simulink model "wlan_phy_rx_pmd"  (Simulink 7.8)
settings: Solver=VariableStepDiscrete  SolverMode=SingleTasking  StartTime=0.0  StopTime=rx_sim_time  RelTol=1e-3
PostLoadFcn:
  | wlan_phy_rx_init
InitFcn:
  | wlan_phy_rx_init
StartFcn:
  | wlan_phy_rx_init
values from neighbouring files (symbols this model uses):
  barker_seq20 = 0.95 * circshift(barker_seq20, [0 4]) ./ max(abs(barker_seq20))    [wlan_phy_rx_init.m]
  bit_scrambler_lfsr_bytes = sum(bit_scrambler_lfsr_bytes .* repmat(2.^[0:7], 127, 1),2)    [wlan_phy_rx_init.m]
  deinterleave_ROM = [deinterleave_ROM interleave_11n_64QAM zeros(1, 512-length(interleave_11n_64QAM))]    [wlan_phy_rx_init.m]
  longCorr_coef_i = [3*real(longCorr_coef)]    [wlan_phy_rx_init.m]
  longCorr_coef_q = [3*imag(longCorr_coef)]    [wlan_phy_rx_init.m]
  MAX_CP_LEN = 32    [wlan_phy_rx_init.m]
  MAX_NCBPS = NCBPS_11n_64QAM    (= 312)    [wlan_phy_rx_init.m]
  MAX_NUM_BYTES = 2^16-1    (= 65535)    [wlan_phy_rx_init.m]
  MAX_NUM_SC = 64    [wlan_phy_rx_init.m]
  MAX_NUM_SYMS = 600    [wlan_phy_rx_init.m]
  NCBPS_11a_BPSK = 48    [wlan_phy_rx_init.m]
  NDBPS_11a_BPSK12 = 24    [wlan_phy_rx_init.m]
  PHY_SIGNAL_MIN_LEN = 14    [wlan_phy_rx_init.m]
  preFFT_sampBuff_numSamps = 4*MAX_NUM_SC    (= 256)    [wlan_phy_rx_init.m]
  rx_sim_sig_samp_time = 8    [wlan_phy_rx_init.m]
  scr_ind_rev = zeros(1,128)    [wlan_phy_rx_init.m]
undefined symbols (not defined in the file or in its folder): gcbh, mcs_rom_11ag, mcs_rom_11n, xlBlockLoadCallback, xlGetNormalizedPeriod, xlipgetpartsetting
libraries: xbsIndex_r4 x3862, simulink x1, xbsEDKLib_r4 x1
(part 12 of 23)

== system wlan_phy_rx_pmd/Sync & Antenna Sel/PHY CCA/S-R Latch3 ==
  S : Inport
  R : Inport  Port=2
  Constant1 : xbsIndex_r4/Constant  const=1  gui_display_data_type=Fixed-point  arith_type=Boolean  n_bits=1  bin_pt=0  preci_type=Single  exp_width=8  frac_width=24  explicit_period=off  period=1  equ=P=C  opselect=C  inp2=PCIN>>17  opr=+  inp1=P  carry=CIN  dbl_ovrd=off  block_type=constant
      settings: block_version=VER_STRING_GOES_HERE
  Convert1 : xbsIndex_r4/Convert  gui_display_data_type=Boolean  arith_type=Boolean  n_bits=1  bin_pt=0  float_type=Single  exp_bits=8  fraction_bits=24  quantization=Truncate  overflow=Wrap  en=off  latency=0  dbl_ovrd=off  pipeline=off  block_type=convert
  Convert2 : xbsIndex_r4/Convert  gui_display_data_type=Boolean  arith_type=Boolean  n_bits=1  bin_pt=0  float_type=Single  exp_bits=8  fraction_bits=24  quantization=Truncate  overflow=Wrap  en=off  latency=0  dbl_ovrd=off  pipeline=off  block_type=convert
  Register2 : xbsIndex_r4/Register  init=0  rst=on  en=on  dbl_ovrd=off  block_type=register
      settings: xl_area=[1 1 0 0 0 0 0]  block_version=VER_STRING_GOES_HERE
  Q : Outport
  wires:
  Constant1/1 -> Register2/1
  R/1 -> Convert1/1
  Register2/1 -> Q/1
  S/1 -> Convert2/1
  Convert1/1 -> Register2/2
  Convert2/1 -> Register2/3

== system wlan_phy_rx_pmd/Sync & Antenna Sel/Pkt Det/DSSS Ant Sel Logic ==
  Det A : Inport
  Det B : Inport  Port=2
  Det C : Inport  Port=3
  Det D : Inport  Port=4
  Concat : xbsIndex_r4/Concat  num_inputs=4  dbl_ovrd=off  block_type=concat
  Logical{,2..4} (4 alike) : xbsIndex_r4/Logical  logical_function=AND  inputs=2  en=off  latency=0  precision=Full  arith_type=Unsigned  n_bits=16  bin_pt=0  align_bp=on  dbl_ovrd=off  block_type=logical
  Logical1 : xbsIndex_r4/Logical  logical_function=OR  inputs=4  en=off  latency=0  precision=Full  arith_type=Unsigned  n_bits=16  bin_pt=0  align_bp=on  dbl_ovrd=off  block_type=logical
  Pkt Det : Outport
  tag scope: PKTDET_STATUS_DSSS=global
  wires:
  Logical4/1 -> Logical1/4, Concat/4
  Logical3/1 -> Logical1/3, Concat/3
  Det D/1 -> Logical4/2
  Det C/1 -> Logical3/2
  [regRx_pktDetEn_RFD] -> Logical4/1
  [regRx_pktDetEn_RFC] -> Logical3/1
  [regRx_pktDetEn_RFB] -> Logical2/1
  Det B/1 -> Logical2/2
  Det A/1 -> Logical/2
  Logical1/1 -> Pkt Det/1
  Logical2/1 -> Logical1/2, Concat/2
  Logical/1 -> Logical1/1, Concat/1
  [regRx_pktDetEn_RFA] -> Logical/1
  Concat/1 -> [PKTDET_STATUS_DSSS]

== system wlan_phy_rx_pmd/Sync & Antenna Sel/Pkt Det/Ext Pkt Det ==
  Reset : Inport
  Constant : Constant  Value=0
  Convert : xbsIndex_r4/Convert  gui_display_data_type=Boolean  arith_type=Boolean  n_bits=16  bin_pt=14  float_type=Single  exp_bits=8  fraction_bits=24  quantization=Truncate  overflow=Wrap  en=off  latency=0  dbl_ovrd=off  pipeline=off  block_type=convert
  Convert1 : xbsIndex_r4/Convert  gui_display_data_type=Boolean  arith_type=Boolean  n_bits=16  bin_pt=14  float_type=Single  exp_bits=8  fraction_bits=24  quantization=Truncate  overflow=Wrap  en=off  latency=0  dbl_ovrd=off  pipeline=off  block_type=convert
  Convert2 : xbsIndex_r4/Convert  gui_display_data_type=Boolean  arith_type=Boolean  n_bits=16  bin_pt=14  float_type=Single  exp_bits=8  fraction_bits=24  quantization=Truncate  overflow=Wrap  en=off  latency=0  dbl_ovrd=off  pipeline=off  block_type=convert
  Debouce : Subsystem
  Logical1 : xbsIndex_r4/Logical  logical_function=AND  inputs=2  en=off  latency=0  precision=Full  arith_type=Unsigned  n_bits=16  bin_pt=0  align_bp=on  dbl_ovrd=off  block_type=logical
  Posedge1 : Subsystem
  Register{1..5} (5 alike) : xbsIndex_r4/Register  init=0  rst=off  en=off  dbl_ovrd=off  block_type=register
  S-R Latch : Subsystem
  pkt_det_in : xbsIndex_r4/Gateway In  gui_display_data_type=Fixed-point  arith_type=Unsigned  n_bits=1  bin_pt=0  preci_type=Single  exp_width=8  frac_width=24  quantization="Round  (unbiased: +/- Inf)"  overflow=Saturate  period=1  dbl_ovrd=off  timing_constraint=None  locs_specified=off  LOCs={}  UseAsADC=off  ADCChannel='1'  inherit_from_input=off  hdl_port=on  block_type=gatewayin
  Det : Outport
  wires:
  Constant/1 -> pkt_det_in/1
  S-R Latch/1(Q) -> Logical1/2
  Posedge1/1(Q) -> Convert1/1
  Reset/1 -> Register5/1
  Debouce/1(Q) -> Posedge1/1(D)
  pkt_det_in/1 -> Register4/1
  Logical1/1 -> Det/1
  [regRx_pktDetEn_Ext] -> Convert2/1
  Convert/1 -> S-R Latch/1(R)
  Convert1/1 -> S-R Latch/2(S)
  Convert2/1 -> Logical1/1
  Register4/1 -> Register1/1
  Register1/1 -> Register2/1
  Register2/1 -> Debouce/1(D)
  Register3/1 -> Debouce/2(R), Convert/1
  Register5/1 -> Register3/1

== system wlan_phy_rx_pmd/Sync & Antenna Sel/Pkt Det/OFDM Ant Sel Logic ==
  Ext Det : Inport
  Det A : Inport  Port=2
  Det B : Inport  Port=3
  Det C : Inport  Port=4
  Det D : Inport  Port=5
  Concat : xbsIndex_r4/Concat  num_inputs=5  dbl_ovrd=off  block_type=concat
  Logical{,2..4} (4 alike) : xbsIndex_r4/Logical  logical_function=AND  inputs=2  en=off  latency=0  precision=Full  arith_type=Unsigned  n_bits=16  bin_pt=0  align_bp=on  dbl_ovrd=off  block_type=logical
  Logical1 : xbsIndex_r4/Logical  logical_function=OR  inputs=4  en=off  latency=0  precision=Full  arith_type=Unsigned  n_bits=16  bin_pt=0  align_bp=on  dbl_ovrd=off  block_type=logical
  Pkt Det : Outport
  tag scope: PKTDET_STATUS_OFDM=global
  wires:
  [regRx_pktDetEn_RFA] -> Logical/1
  Logical/1 -> Logical1/1, Concat/5
  Logical2/1 -> Logical1/2, Concat/4
  Logical1/1 -> Pkt Det/1
  Det A/1 -> Logical/2
  Det B/1 -> Logical2/2
  [regRx_pktDetEn_RFB] -> Logical2/1
  [regRx_pktDetEn_RFC] -> Logical3/1
  [regRx_pktDetEn_RFD] -> Logical4/1
  Det C/1 -> Logical3/2
  Det D/1 -> Logical4/2
  Logical3/1 -> Logical1/3, Concat/3
  Logical4/1 -> Logical1/4, Concat/2
  Concat/1 -> [PKTDET_STATUS_OFDM]
  Ext Det/1 -> Concat/1

== system wlan_phy_rx_pmd/Sync & Antenna Sel/Pkt Det/RF A Det ==
  Reset OFDM : Inport
  Reset DSSS : Inport  Port=2
  Rx I : Inport  Port=3
  Rx Q : Inport  Port=4
  IQ Valid : Inport  Port=5
  RSSI : Inport  Port=6
  Auto-Corr Pkt Det : Subsystem
  Logical : xbsIndex_r4/Logical  logical_function=OR  inputs=2  en=off  latency=0  precision=Full  arith_type=Unsigned  n_bits=16  bin_pt=0  align_bp=on  dbl_ovrd=off  block_type=logical
  Logical1 : xbsIndex_r4/Logical  logical_function=OR  inputs=2  en=off  latency=0  precision=Full  arith_type=Unsigned  n_bits=16  bin_pt=0  align_bp=on  dbl_ovrd=off  block_type=logical
  Logical2 : xbsIndex_r4/Logical  logical_function=AND  inputs=2  en=off  latency=0  precision=Full  arith_type=Unsigned  n_bits=16  bin_pt=0  align_bp=on  dbl_ovrd=off  block_type=logical
  Logical3 : xbsIndex_r4/Logical  logical_function=AND  inputs=2  en=off  latency=0  precision=Full  arith_type=Unsigned  n_bits=16  bin_pt=0  align_bp=on  dbl_ovrd=off  block_type=logical
  Mux : xbsIndex_r4/Mux  inputs=2  en=off  latency=0  precision=Full  arith_type=Unsigned  n_bits=16  bin_pt=14  quantization=Truncate  overflow=Wrap  dbl_ovrd=off  block_type=mux
  Mux1 : xbsIndex_r4/Mux  inputs=2  en=off  latency=0  precision=Full  arith_type=Unsigned  n_bits=16  bin_pt=14  quantization=Truncate  overflow=Wrap  dbl_ovrd=off  block_type=mux
  RSSI Pkt Det : Subsystem
  Det OFDM : Outport
  Det DSSS : Outport  Port=2
  wires:
  Logical/1 -> Mux/2
  IQ Valid/1 -> Auto-Corr Pkt Det/5(rx_valid)
  Rx Q/1 -> Auto-Corr Pkt Det/4(rx_Q)
  Rx I/1 -> Auto-Corr Pkt Det/3(rx_I)
  Logical1/1 -> Mux1/2
  Reset OFDM/1 -> Auto-Corr Pkt Det/1(Reset OFDM), RSSI Pkt Det/1(Reset OFDM)
  Reset DSSS/1 -> Auto-Corr Pkt Det/2(Reset DSSS), RSSI Pkt Det/2(Reset DSSS)
  [regRx_pktDet_requireBothA] -> Mux/1, Mux1/1
  Logical3/1 -> Mux/3
  Mux/1 -> Det OFDM/1
  RSSI/1 -> RSSI Pkt Det/3(Avg RSSI)
  Auto-Corr Pkt Det/2(Det DSSS) -> Logical1/1, Logical2/1
  RSSI Pkt Det/2(PktDet DSSS) -> Logical1/2, Logical2/2
  Auto-Corr Pkt Det/1(Det OFDM) -> Logical/1, Logical3/1
  RSSI Pkt Det/1(PktDet OFDM) -> Logical/2, Logical3/2
  Logical2/1 -> Mux1/3
  Mux1/1 -> Det DSSS/1

== system wlan_phy_rx_pmd/Sync & Antenna Sel/Pkt Det/RF B Det ==
  Reset OFDM : Inport
  Reset DSSS : Inport  Port=2
  Rx I : Inport  Port=3
  Rx Q : Inport  Port=4
  IQ Valid : Inport  Port=5
  RSSI : Inport  Port=6
  Auto-Corr Pkt Det : Subsystem
  Logical : xbsIndex_r4/Logical  logical_function=OR  inputs=2  en=off  latency=0  precision=Full  arith_type=Unsigned  n_bits=16  bin_pt=0  align_bp=on  dbl_ovrd=off  block_type=logical
  Logical1 : xbsIndex_r4/Logical  logical_function=OR  inputs=2  en=off  latency=0  precision=Full  arith_type=Unsigned  n_bits=16  bin_pt=0  align_bp=on  dbl_ovrd=off  block_type=logical
  Logical2 : xbsIndex_r4/Logical  logical_function=AND  inputs=2  en=off  latency=0  precision=Full  arith_type=Unsigned  n_bits=16  bin_pt=0  align_bp=on  dbl_ovrd=off  block_type=logical
  Logical3 : xbsIndex_r4/Logical  logical_function=AND  inputs=2  en=off  latency=0  precision=Full  arith_type=Unsigned  n_bits=16  bin_pt=0  align_bp=on  dbl_ovrd=off  block_type=logical
  Mux : xbsIndex_r4/Mux  inputs=2  en=off  latency=0  precision=Full  arith_type=Unsigned  n_bits=16  bin_pt=14  quantization=Truncate  overflow=Wrap  dbl_ovrd=off  block_type=mux
  Mux1 : xbsIndex_r4/Mux  inputs=2  en=off  latency=0  precision=Full  arith_type=Unsigned  n_bits=16  bin_pt=14  quantization=Truncate  overflow=Wrap  dbl_ovrd=off  block_type=mux
  RSSI Pkt Det : Subsystem
  Det OFDM : Outport
  Det DSSS : Outport  Port=2
  wires:
  RSSI Pkt Det/1(PktDet OFDM) -> Logical3/2, Logical/2
  Auto-Corr Pkt Det/1(Det OFDM) -> Logical3/1, Logical/1
  RSSI Pkt Det/2(PktDet DSSS) -> Logical1/2, Logical2/2
  Auto-Corr Pkt Det/2(Det DSSS) -> Logical1/1, Logical2/1
  RSSI/1 -> RSSI Pkt Det/3(Avg RSSI)
  Mux/1 -> Det OFDM/1
  Logical3/1 -> Mux/3
  [regRx_pktDet_requireBothB] -> Mux/1, Mux1/1
  Reset DSSS/1 -> RSSI Pkt Det/2(Reset DSSS), Auto-Corr Pkt Det/2(Reset DSSS)
  Reset OFDM/1 -> RSSI Pkt Det/1(Reset OFDM), Auto-Corr Pkt Det/1(Reset OFDM)
  Rx I/1 -> Auto-Corr Pkt Det/3(rx_I)
  Rx Q/1 -> Auto-Corr Pkt Det/4(rx_Q)
  IQ Valid/1 -> Auto-Corr Pkt Det/5(rx_valid)
  Logical/1 -> Mux/2
  Mux1/1 -> Det DSSS/1
  Logical1/1 -> Mux1/2
  Logical2/1 -> Mux1/3

== system wlan_phy_rx_pmd/Sync & Antenna Sel/Resets/Negedge ==
  D : Inport
  Delay : xbsIndex_r4/Delay  rst=off  infoeditControl=Selection of Reset will increase slice count due to use of real FFs and instead of SRLs  en=off  latency=1  dbl_ovrd=off  reg_retiming=off  block_type=delay
      settings: infoedit=Hardware notes: A delay line is a chain, each link of which is an SRL16 followed by a flip-flop. If register retiming is enabled, the delay line is a chain of flip-flops.  xl_area=[1 1 0 0 0 0 0]  block_version=VER_STRING_GOES_HERE
  Inverter : xbsIndex_r4/Inverter  en=off  latency=0  dbl_ovrd=off  block_type=inv
      settings: xl_area=[0 0 0 0 0 0 0]  block_version=VER_STRING_GOES_HERE
  Logical1 : xbsIndex_r4/Logical  logical_function=AND  inputs=2  en=off  latency=0  precision=Full  arith_type=Unsigned  n_bits=8  bin_pt=2  align_bp=on  dbl_ovrd=off  block_type=logical
      settings: xl_area=[1 0 0 1 0 0 0]  block_version=VER_STRING_GOES_HERE
  Q : Outport
  wires:
  D/1 -> Delay/1, Inverter/1
  Logical1/1 -> Q/1
  Delay/1 -> Logical1/1
  Inverter/1 -> Logical1/2

== system wlan_phy_rx_pmd/Sync & Antenna Sel/Resets/Reset Gen ==
  note: Extend pkt det reset by programmable
      | duration, to avoid false positives after
      | actual receptions due to Rx transients
      | during AGC reset
  Done : Inport
  Concat : xbsIndex_r4/Concat  num_inputs=2  dbl_ovrd=off  block_type=concat
  Constant : xbsIndex_r4/Constant  const=0  gui_display_data_type=Fixed-point  arith_type=Unsigned  n_bits=4  bin_pt=0  preci_type=Single  exp_width=8  frac_width=24  explicit_period=off  period=1  equ=P=C  opselect=C  inp2=PCIN>>17  opr=+  inp1=P  carry=CIN  dbl_ovrd=off  block_type=constant
  Counter1 : xbsIndex_r4/Counter  cnt_type=Free Running  cnt_to=Inf  operation=Up  start_count=0  cnt_by_val=1  arith_type=Unsigned  n_bits=10  bin_pt=0  load_pin=off  rst=on  en=on  explicit_period=off  period=4  dbl_ovrd=off  use_behavioral_HDL=off  implementation=Fabric  use_rpm=off  block_type=counter
  Inverter : xbsIndex_r4/Inverter  en=off  latency=0  dbl_ovrd=off  block_type=inv
      settings: xl_area=[0 0 0 0 0 0 0]  block_version=VER_STRING_GOES_HERE
  Logical2 : xbsIndex_r4/Logical  logical_function=OR  inputs=3  en=off  latency=0  precision=Full  arith_type=Unsigned  n_bits=16  bin_pt=0  align_bp=on  dbl_ovrd=off  block_type=logical
  Register : xbsIndex_r4/Register  init=0  rst=off  en=off  dbl_ovrd=off  block_type=register
  Relational3 : xbsIndex_r4/Relational  mode=a>b  en=off  latency=0  dbl_ovrd=off  block_type=relational
      settings: block_version=VER_STRING_GOES_HERE
  S-R Latch2 : Subsystem
  negedge : Subsystem
  Reset : Outport
  wires:
  Done/1 -> Logical2/2, negedge/1(D)
  negedge/1(Q) -> S-R Latch2/1(S), Logical2/3
  S-R Latch2/1(Q) -> Counter1/2, Logical2/1, Inverter/1
  Counter1/1 -> Relational3/1
  Relational3/1 -> S-R Latch2/2(R)
  Logical2/1 -> Reset/1
  Concat/1 -> Relational3/2
  Constant/1 -> Concat/2
  [regRx_PKT_DET_RESET_EXT_DUR] -> Register/1
  Register/1 -> Concat/1
  Inverter/1 -> Counter1/1

== system wlan_phy_rx_pmd/Sync & Antenna Sel/Resets/Reset Gen1 ==
  note: Extend pkt det reset by programmable
      | duration, to avoid false positives after
      | actual receptions due to Rx transients
      | during AGC reset
  Done : Inport
  Concat : xbsIndex_r4/Concat  num_inputs=2  dbl_ovrd=off  block_type=concat
  Constant : xbsIndex_r4/Constant  const=0  gui_display_data_type=Fixed-point  arith_type=Unsigned  n_bits=4  bin_pt=0  preci_type=Single  exp_width=8  frac_width=24  explicit_period=off  period=1  equ=P=C  opselect=C  inp2=PCIN>>17  opr=+  inp1=P  carry=CIN  dbl_ovrd=off  block_type=constant
  Counter1 : xbsIndex_r4/Counter  cnt_type=Free Running  cnt_to=Inf  operation=Up  start_count=0  cnt_by_val=1  arith_type=Unsigned  n_bits=10  bin_pt=0  load_pin=off  rst=on  en=on  explicit_period=off  period=4  dbl_ovrd=off  use_behavioral_HDL=off  implementation=Fabric  use_rpm=off  block_type=counter
  Inverter : xbsIndex_r4/Inverter  en=off  latency=0  dbl_ovrd=off  block_type=inv
      settings: xl_area=[0 0 0 0 0 0 0]  block_version=VER_STRING_GOES_HERE
  Logical2 : xbsIndex_r4/Logical  logical_function=OR  inputs=3  en=off  latency=0  precision=Full  arith_type=Unsigned  n_bits=16  bin_pt=0  align_bp=on  dbl_ovrd=off  block_type=logical
  Register : xbsIndex_r4/Register  init=0  rst=off  en=off  dbl_ovrd=off  block_type=register
  Relational3 : xbsIndex_r4/Relational  mode=a>b  en=off  latency=0  dbl_ovrd=off  block_type=relational
      settings: block_version=VER_STRING_GOES_HERE
  S-R Latch2 : Subsystem
  negedge : Subsystem
  Reset : Outport
  wires:
  Inverter/1 -> Counter1/1
  Register/1 -> Concat/1
  [regRx_PKT_DET_RESET_EXT_DUR] -> Register/1
  Constant/1 -> Concat/2
  Concat/1 -> Relational3/2
  Logical2/1 -> Reset/1
  Relational3/1 -> S-R Latch2/2(R)
  Counter1/1 -> Relational3/1
  S-R Latch2/1(Q) -> Inverter/1, Logical2/1, Counter1/2
  negedge/1(Q) -> Logical2/3, S-R Latch2/1(S)
  Done/1 -> negedge/1(D), Logical2/2

== system wlan_phy_rx_pmd/CFO & Samp Buffer/CFO Correction/Sim Reset/Subsystem ==
  Disregard Subsystem : xbsIndex_r4/Disregard Subsystem  Tag=discardX  block_type=disregard
  Gateway In : xbsIndex_r4/Gateway In  gui_display_data_type=Boolean  arith_type=Boolean  n_bits=16  bin_pt=14  preci_type=Single  exp_width=8  frac_width=24  quantization="Round  (unbiased: +/- Inf)"  overflow=Saturate  period=1  dbl_ovrd=off  timing_constraint=None  locs_specified=off  LOCs={}  UseAsADC=off  ADCChannel='1'  inherit_from_input=off  hdl_port=on  block_type=gatewayin
  Pulse Generator : DiscretePulseGenerator  Period=1e9  PulseWidth=16  PhaseDelay=1
  Out1 : Outport
  wires:
  Pulse Generator/1 -> Gateway In/1
  Gateway In/1 -> Out1/1

== system wlan_phy_rx_pmd/CFO & Samp Buffer/Samp Buffer/FFT Load Ctrl/Count-To Calc ==
  Skip Sel : Inport
  AddSub1 : xbsIndex_r4/AddSub  mode=Addition  use_carryin=off  use_carryout=off  en=off  latency=0  precision=User Defined  arith_type=Unsigned  n_bits=ceil(log2(preFFT_sampBuff_numSamps))  bin_pt=0  quantization=Truncate  overflow=Wrap  dbl_ovrd=off  use_behavioral_HDL=off  hw_selection=Fabric  pipelined=off  block_type=addsub
  Constant4 : xbsIndex_r4/Constant  const=0  gui_display_data_type=Fixed-point  arith_type=Unsigned  n_bits=1  bin_pt=0  preci_type=Single  exp_width=8  frac_width=24  explicit_period=off  period=8  equ=P=C  opselect=C  inp2=PCIN>>17  opr=+  inp1=P  carry=CIN  dbl_ovrd=off  block_type=constant
  Display : Display
  Display1 : Display
  Gateway Out : xbsIndex_r4/Gateway Out  inherit_from_input=off  hdl_port=off  timing_constraint=None  locs_specified=off  LOCs={}  UseAsDAC=off  DACChannel='1'  block_type=gatewayout
  Gateway Out1 : xbsIndex_r4/Gateway Out  inherit_from_input=off  hdl_port=off  timing_constraint=None  locs_specified=off  LOCs={}  UseAsDAC=off  DACChannel='1'  block_type=gatewayout
  Mux : xbsIndex_r4/Mux  inputs=2  en=off  latency=0  precision=Full  arith_type=Unsigned  n_bits=16  bin_pt=14  quantization=Truncate  overflow=Wrap  dbl_ovrd=off  block_type=mux
  Samp+Skip : Outport
  Samp : Outport  Port=2
  wires:
  [regRx_CP_LEN] -> Mux/3, Gateway Out/1
  Skip Sel/1 -> Mux/1
  Constant4/1 -> Mux/2
  Mux/1 -> AddSub1/1
  AddSub1/1 -> Samp+Skip/1
  [regRx_NUM_SC] -> AddSub1/2, Samp/1, Gateway Out1/1
  Gateway Out/1 -> Display/1
  Gateway Out1/1 -> Display1/1

== system wlan_phy_rx_pmd/CFO & Samp Buffer/Samp Buffer/FFT Load Ctrl/First Samp Calc ==
  Wr Addr : Inport
  LTS Sync : Inport  Port=2
  AddSub2 : xbsIndex_r4/AddSub  mode=Subtraction  use_carryin=off  use_carryout=off  en=off  latency=0  precision=User Defined  arith_type=Unsigned  n_bits=ceil(log2(preFFT_sampBuff_numSamps))  bin_pt=0  quantization=Truncate  overflow=Wrap  dbl_ovrd=off  use_behavioral_HDL=off  hw_selection=Fabric  pipelined=off  block_type=addsub
  AddSub3 : xbsIndex_r4/AddSub  mode=Addition  use_carryin=off  use_carryout=off  en=off  latency=0  precision=User Defined  arith_type=Unsigned  n_bits=ceil(log2(preFFT_sampBuff_numSamps))  bin_pt=0  quantization=Truncate  overflow=Wrap  dbl_ovrd=off  use_behavioral_HDL=off  hw_selection=Fabric  pipelined=off  block_type=addsub
  Register : xbsIndex_r4/Register  init=0  rst=off  en=on  dbl_ovrd=off  block_type=register
  Shift : xbsIndex_r4/Shift  shift_dir=Left  shift_bits=1  en=off  latency=0  precision=Full  arith_type="Signed  (2's comp)"  n_bits=16  bin_pt=14  quantization=Truncate  overflow=Wrap  dbl_ovrd=off  block_type=shift
  Samp Addr : Outport
  wires:
  [regRx_NUM_SC] -> Shift/1
  Shift/1 -> AddSub3/1
  AddSub3/1 -> AddSub2/2
  Register/1 -> AddSub2/1
  Wr Addr/1 -> Register/1
  AddSub2/1 -> Samp Addr/1
  LTS Sync/1 -> Register/2
  [regRx_FFT_OFFSET] -> AddSub3/2

== system wlan_phy_rx_pmd/CFO & Samp Buffer/Samp Buffer/FFT Load Ctrl/Posedge ==
  note: Rising Edge Detection
  D : Inport
  Delay : xbsIndex_r4/Delay  rst=off  infoeditControl=Selection of Reset will increase slice count due to use of real FFs and instead of SRLs  en=off  latency=1  dbl_ovrd=off  reg_retiming=off  block_type=delay
      settings: infoedit=Hardware notes: A delay line is a chain, each link of which is an SRL16 followed by a flip-flop. If register retiming is enabled, the delay line is a chain of flip-flops.  xl_area=[1 1 0 0 0 0 0]  block_version=VER_STRING_GOES_HERE
  Inverter : xbsIndex_r4/Inverter  en=off  latency=0  dbl_ovrd=off  block_type=inv
      settings: xl_area=[0 0 0 0 0 0 0]  block_version=VER_STRING_GOES_HERE
  Logical1 : xbsIndex_r4/Logical  logical_function=AND  inputs=2  en=off  latency=0  precision=Full  arith_type=Unsigned  n_bits=8  bin_pt=2  align_bp=on  dbl_ovrd=off  block_type=logical
      settings: xl_area=[1 0 0 1 0 0 0]  block_version=VER_STRING_GOES_HERE
  Q : Outport
  wires:
  D/1 -> Logical1/2, Delay/1
  Logical1/1 -> Q/1
  Inverter/1 -> Logical1/1
  Delay/1 -> Inverter/1

== system wlan_phy_rx_pmd/CFO & Samp Buffer/Samp Buffer/FFT Load Ctrl/S-R Latch1 ==
  S : Inport
  R : Inport  Port=2
  Constant1 : xbsIndex_r4/Constant  const=1  gui_display_data_type=Fixed-point  arith_type=Boolean  n_bits=1  bin_pt=0  preci_type=Single  exp_width=8  frac_width=24  explicit_period=off  period=1  equ=P=C  opselect=C  inp2=PCIN>>17  opr=+  inp1=P  carry=CIN  dbl_ovrd=off  block_type=constant
      settings: block_version=VER_STRING_GOES_HERE
  Convert1 : xbsIndex_r4/Convert  gui_display_data_type=Boolean  arith_type=Boolean  n_bits=1  bin_pt=0  float_type=Single  exp_bits=8  fraction_bits=24  quantization=Truncate  overflow=Wrap  en=off  latency=0  dbl_ovrd=off  pipeline=off  block_type=convert
  Convert2 : xbsIndex_r4/Convert  gui_display_data_type=Boolean  arith_type=Boolean  n_bits=1  bin_pt=0  float_type=Single  exp_bits=8  fraction_bits=24  quantization=Truncate  overflow=Wrap  en=off  latency=0  dbl_ovrd=off  pipeline=off  block_type=convert
  Register2 : xbsIndex_r4/Register  init=0  rst=on  en=on  dbl_ovrd=off  block_type=register
      settings: xl_area=[1 1 0 0 0 0 0]  block_version=VER_STRING_GOES_HERE
  Q : Outport
  wires:
  S/1 -> Convert2/1
  Register2/1 -> Q/1
  R/1 -> Convert1/1
  Constant1/1 -> Register2/1
  Convert1/1 -> Register2/2
  Convert2/1 -> Register2/3

== system wlan_phy_rx_pmd/Chan Est & EQ/Equalizer, Pilot Processing & PHY Mode Det/PHY Mode Det/Mod Det ==
  note: PHY Mode Det calc = sum(abs(I) - abs(Q)):
      |   Large Positive => BPSK
      |   Large Negative => QBPSK
      |   Near Zero => QAM
  I : Inport
  Q : Inport  Port=2
  " BPSK" : xbsIndex_r4/Logical  logical_function=AND  inputs=2  en=off  latency=0  precision=Full  arith_type=Unsigned  n_bits=16  bin_pt=0  align_bp=on  dbl_ovrd=off  block_type=logical
  " QAM" : xbsIndex_r4/Inverter  en=off  latency=0  dbl_ovrd=off  block_type=inv
  " QBPSK" : xbsIndex_r4/Logical  logical_function=AND  inputs=2  en=off  latency=0  precision=Full  arith_type=Unsigned  n_bits=16  bin_pt=0  align_bp=on  dbl_ovrd=off  block_type=logical
  Absolute2 : xbsIndex_r4/Absolute  precision=Full  arith_type="Signed  (2's comp)"  n_bits=8  bin_pt=7  quantization=Truncate  overflow=Saturate  en=off  latency=0  dbl_ovrd=off  block_type=abs
  AddSub : xbsIndex_r4/AddSub  mode=Subtraction  use_carryin=off  use_carryout=off  en=off  latency=0  precision=Full  arith_type=Unsigned  n_bits=16  bin_pt=14  quantization=Truncate  overflow=Wrap  dbl_ovrd=off  use_behavioral_HDL=off  hw_selection=Fabric  pipelined=off  block_type=addsub
  Constant5 : xbsIndex_r4/Constant  const=0  gui_display_data_type=Fixed-point  arith_type=Unsigned  n_bits=2  bin_pt=0  preci_type=Single  exp_width=8  frac_width=24  explicit_period=off  period=1  equ=P=C  opselect=C  inp2=PCIN>>17  opr=+  inp1=P  carry=CIN  dbl_ovrd=off  block_type=constant
  Constant6 : xbsIndex_r4/Constant  const=20  gui_display_data_type=Fixed-point  arith_type=Unsigned  n_bits=5  bin_pt=0  preci_type=Single  exp_width=8  frac_width=24  explicit_period=off  period=1  equ=P=C  opselect=C  inp2=PCIN>>17  opr=+  inp1=P  carry=CIN  dbl_ovrd=off  block_type=constant
  Inverter : xbsIndex_r4/Inverter  en=off  latency=0  dbl_ovrd=off  block_type=inv
  Relational4 : xbsIndex_r4/Relational  mode=a>b  en=off  latency=1  dbl_ovrd=off  block_type=relational
  Relational5 : xbsIndex_r4/Relational  mode=a>b  en=off  latency=1  dbl_ovrd=off  block_type=relational
  QAM : Outport
  QBPSK : Outport  Port=2
  BPSK : Outport  Port=3
  wires:
  Constant5/1 -> Relational4/2
  AddSub/1 -> Relational4/1, Absolute2/1
  Absolute2/1 -> Relational5/1
  Constant6/1 -> Relational5/2
  Relational5/1 -> " BPSK"/1, " QBPSK"/1, " QAM"/1
  Relational4/1 -> " BPSK"/2, Inverter/1
  Inverter/1 -> " QBPSK"/2
  " BPSK"/1 -> BPSK/1
  I/1 -> AddSub/1
  Q/1 -> AddSub/2
  " QBPSK"/1 -> QBPSK/1
  " QAM"/1 -> QAM/1

== system wlan_phy_rx_pmd/Chan Est & EQ/Equalizer, Pilot Processing & PHY Mode Det/PHY Mode Det/edge ==
  D : Inport
  Delay6 : xbsIndex_r4/Delay  rst=off  infoeditControl=Selection of Reset will increase slice count due to use of real FFs and instead of SRLs  en=off  latency=1  dbl_ovrd=off  reg_retiming=off  block_type=delay
  Logical : xbsIndex_r4/Logical  logical_function=XOR  inputs=2  en=off  latency=0  precision=Full  arith_type=Unsigned  n_bits=16  bin_pt=0  align_bp=on  dbl_ovrd=off  block_type=logical
  Q : Outport
  wires:
  Delay6/1 -> Logical/2
  D/1 -> Logical/1, Delay6/1
  Logical/1 -> Q/1

== system wlan_phy_rx_pmd/Chan Est & EQ/Equalizer, Pilot Processing & PHY Mode Det/PHY Mode Det/negedge ==
  D : Inport
  Delay6 : xbsIndex_r4/Delay  rst=off  infoeditControl=Selection of Reset will increase slice count due to use of real FFs and instead of SRLs  en=off  latency=1  dbl_ovrd=off  reg_retiming=off  block_type=delay
  Inverter : xbsIndex_r4/Inverter  en=off  latency=0  dbl_ovrd=off  block_type=inv
  Logical : xbsIndex_r4/Logical  logical_function=AND  inputs=2  en=off  latency=0  precision=Full  arith_type=Unsigned  n_bits=16  bin_pt=0  align_bp=on  dbl_ovrd=off  block_type=logical
  Q : Outport
  wires:
  Delay6/1 -> Logical/2
  D/1 -> Delay6/1, Inverter/1
  Logical/1 -> Q/1
  Inverter/1 -> Logical/1

== system wlan_phy_rx_pmd/Chan Est & EQ/Equalizer, Pilot Processing & PHY Mode Det/PHY Mode Det/negedge1 ==
  D : Inport
  Delay6 : xbsIndex_r4/Delay  rst=off  infoeditControl=Selection of Reset will increase slice count due to use of real FFs and instead of SRLs  en=off  latency=1  dbl_ovrd=off  reg_retiming=off  block_type=delay
  Inverter : xbsIndex_r4/Inverter  en=off  latency=0  dbl_ovrd=off  block_type=inv
  Logical : xbsIndex_r4/Logical  logical_function=AND  inputs=2  en=off  latency=0  precision=Full  arith_type=Unsigned  n_bits=16  bin_pt=0  align_bp=on  dbl_ovrd=off  block_type=logical
  Q : Outport
  wires:
  Inverter/1 -> Logical/1
  Logical/1 -> Q/1
  D/1 -> Inverter/1, Delay6/1
  Delay6/1 -> Logical/2

== system wlan_phy_rx_pmd/Chan Est & EQ/Equalizer, Pilot Processing & PHY Mode Det/Phase Err Calc/Accum1 ==
  note: Pilot tones must be spaced by more subcarriers
      | than latency of this addr. With natural order FFT
      | outputs worst case is 7->21 = 14 cycles
  En : Inport
  D : Inport  Port=2
  Rst : Inport  Port=3
  AddSub : xbsIndex_r4/AddSub  mode=Addition  use_carryin=off  use_carryout=off  en=on  latency=4  precision=Full  arith_type=Unsigned  n_bits=16  bin_pt=14  quantization=Truncate  overflow=Wrap  dbl_ovrd=off  use_behavioral_HDL=off  hw_selection=DSP48  pipelined=off  block_type=addsub
  Delay5 : xbsIndex_r4/Delay  rst=off  infoeditControl=Selection of Reset will increase slice count due to use of real FFs and instead of SRLs  en=off  latency=4  dbl_ovrd=off  reg_retiming=off  block_type=delay
  Register : xbsIndex_r4/Register  init=0  rst=on  en=on  dbl_ovrd=off  block_type=register
  Sum : Outport
  wires:
  Delay5/1 -> Register/3
  AddSub/1 -> Register/1, Sum/1
  En/1 -> Delay5/1, AddSub/3
  Rst/1 -> Register/2
  D/1 -> AddSub/1
  Register/1 -> AddSub/2

== system wlan_phy_rx_pmd/Chan Est & EQ/Equalizer, Pilot Processing & PHY Mode Det/Phase Err Calc/Accum2 ==
  note: Pilot tones must be spaced by more subcarriers
      | than latency of this addr. With natural order FFT
      | outputs worst case is 7->21 = 14 cycles
  En : Inport
  D : Inport  Port=2
  Rst : Inport  Port=3
  AddSub : xbsIndex_r4/AddSub  mode=Addition  use_carryin=off  use_carryout=off  en=on  latency=4  precision=Full  arith_type=Unsigned  n_bits=16  bin_pt=14  quantization=Truncate  overflow=Wrap  dbl_ovrd=off  use_behavioral_HDL=off  hw_selection=DSP48  pipelined=off  block_type=addsub
  Delay5 : xbsIndex_r4/Delay  rst=off  infoeditControl=Selection of Reset will increase slice count due to use of real FFs and instead of SRLs  en=off  latency=4  dbl_ovrd=off  reg_retiming=off  block_type=delay
  Register : xbsIndex_r4/Register  init=0  rst=on  en=on  dbl_ovrd=off  block_type=register
  Sum : Outport
  wires:
  Register/1 -> AddSub/2
  D/1 -> AddSub/1
  Rst/1 -> Register/2
  En/1 -> AddSub/3, Delay5/1
  AddSub/1 -> Sum/1, Register/1
  Delay5/1 -> Register/3

== system wlan_phy_rx_pmd/Chan Est & EQ/Equalizer, Pilot Processing & PHY Mode Det/Phase Err Calc/Count ==
  note: Latency to match phase accum latency
  En : Inport
  Rst : Inport  Port=2
  Constant3 : xbsIndex_r4/Constant  const=4  gui_display_data_type=Fixed-point  arith_type=Unsigned  n_bits=6  bin_pt=0  preci_type=Single  exp_width=8  frac_width=24  explicit_period=off  period=8  equ=P=C  opselect=C  inp2=PCIN>>17  opr=+  inp1=P  carry=CIN  dbl_ovrd=off  block_type=constant
  Counter : xbsIndex_r4/Counter  cnt_type=Free Running  cnt_to=Inf  operation=Up  start_count=0  cnt_by_val=1  arith_type=Unsigned  n_bits=3  bin_pt=0  load_pin=off  rst=on  en=on  explicit_period=off  period=1  dbl_ovrd=off  use_behavioral_HDL=off  implementation=Fabric  block_type=counter
  Delay : xbsIndex_r4/Delay  rst=off  infoeditControl=Selection of Reset will increase slice count due to use of real FFs and instead of SRLs  en=off  latency=1  dbl_ovrd=off  reg_retiming=off  block_type=delay
  Delay1 : xbsIndex_r4/Delay  rst=off  infoeditControl=Selection of Reset will increase slice count due to use of real FFs and instead of SRLs  en=off  latency=4  dbl_ovrd=off  reg_retiming=off  block_type=delay
  Logical : xbsIndex_r4/Logical  logical_function=AND  inputs=2  en=off  latency=0  precision=Full  arith_type=Unsigned  n_bits=16  bin_pt=0  align_bp=on  dbl_ovrd=off  block_type=logical
  Relational7 : xbsIndex_r4/Relational  mode=a=b  en=off  latency=0  dbl_ovrd=off  block_type=relational
      settings: xl_area=[2 0 0 3 0 0 0]  block_version=VER_STRING_GOES_HERE
  Last : Outport
  wires:
  Counter/1 -> Relational7/2
  Constant3/1 -> Relational7/1
  Relational7/1 -> Logical/1
  En/1 -> Counter/2, Delay/1
  Rst/1 -> Counter/1
  Logical/1 -> Delay1/1
  Delay/1 -> Logical/2
  Delay1/1 -> Last/1

== system wlan_phy_rx_pmd/Chan Est & EQ/Equalizer, Pilot Processing & PHY Mode Det/Phase Err Calc/Descramble ==
  note: Match latency of edge det
      | on sym index
  note: Advance state of pilot scrambling LFSR on all
      | OFDM symbols *except* HT-SFT/HT-LTF in
      | symbols 5,6
  Negate : Inport
  x_I : Inport  Port=2
  x_Q : Inport  Port=3
  Sym Ind : Inport  Port=4
  Constant1 : xbsIndex_r4/Constant  const=5  gui_display_data_type=Fixed-point  arith_type=Unsigned  n_bits=3  bin_pt=0  preci_type=Single  exp_width=8  frac_width=24  explicit_period=off  period=1  equ=P=C  opselect=C  inp2=PCIN>>17  opr=+  inp1=P  carry=CIN  dbl_ovrd=off  block_type=constant
  Constant2 : xbsIndex_r4/Constant  const=3  gui_display_data_type=Fixed-point  arith_type=Unsigned  n_bits=2  bin_pt=0  preci_type=Single  exp_width=8  frac_width=24  explicit_period=off  period=8  equ=P=C  opselect=C  inp2=PCIN>>17  opr=+  inp1=P  carry=CIN  dbl_ovrd=off  block_type=constant
  Constant3 : xbsIndex_r4/Constant  const=6  gui_display_data_type=Fixed-point  arith_type=Unsigned  n_bits=3  bin_pt=0  preci_type=Single  exp_width=8  frac_width=24  explicit_period=off  period=1  equ=P=C  opselect=C  inp2=PCIN>>17  opr=+  inp1=P  carry=CIN  dbl_ovrd=off  block_type=constant
  Delay{,1..3} (4 alike) : xbsIndex_r4/Delay  rst=off  infoeditControl=Selection of Reset will increase slice count due to use of real FFs and instead of SRLs  en=off  latency=1  dbl_ovrd=off  reg_retiming=off  block_type=delay
  Logical : xbsIndex_r4/Logical  logical_function=XOR  inputs=2  en=off  latency=0  precision=Full  arith_type=Unsigned  n_bits=8  bin_pt=2  align_bp=on  dbl_ovrd=off  block_type=logical
      settings: xl_area=[0, 0, 0, 0, 0, 0, 0]  block_version=VER_STRING_GOES_HERE
  Logical1 : xbsIndex_r4/Logical  logical_function=OR  inputs=2  en=off  latency=0  precision=Full  arith_type=Unsigned  n_bits=16  bin_pt=0  align_bp=on  dbl_ovrd=off  block_type=logical
  Logical2 : xbsIndex_r4/Logical  logical_function=AND  inputs=2  en=off  latency=0  precision=Full  arith_type=Unsigned  n_bits=16  bin_pt=0  align_bp=on  dbl_ovrd=off  block_type=logical
  Logical4 : xbsIndex_r4/Logical  logical_function=NAND  inputs=2  en=off  latency=0  precision=Full  arith_type=Unsigned  n_bits=16  bin_pt=0  align_bp=on  dbl_ovrd=off  block_type=logical
  Mux1 : xbsIndex_r4/Mux  inputs=2  en=off  latency=0  precision=Full  arith_type="Signed  (2's comp)"  n_bits=8  bin_pt=2  quantization=Truncate  overflow=Wrap  dbl_ovrd=off  block_type=mux
      settings: xl_area=[16 0 0 32 0 0 0]  block_version=VER_STRING_GOES_HERE
  Mux2 : xbsIndex_r4/Mux  inputs=2  en=off  latency=0  precision=Full  arith_type="Signed  (2's comp)"  n_bits=8  bin_pt=2  quantization=Truncate  overflow=Wrap  dbl_ovrd=off  block_type=mux
      settings: xl_area=[16 0 0 32 0 0 0]  block_version=VER_STRING_GOES_HERE
  Negate1 : xbsIndex_r4/Negate  precision=Full  arith_type="Signed  (2's comp)"  n_bits=17  bin_pt=15  quantization="Round  (unbiased: +/- Inf)"  overflow=Saturate  en=off  latency=1  dbl_ovrd=off  block_type=negate
      settings: block_version=VER_STRING_GOES_HERE
  Negate2 : xbsIndex_r4/Negate  precision=Full  arith_type="Signed  (2's comp)"  n_bits=17  bin_pt=15  quantization="Round  (unbiased: +/- Inf)"  overflow=Saturate  en=off  latency=1  dbl_ovrd=off  block_type=negate
      settings: block_version=VER_STRING_GOES_HERE
  Relational1 : xbsIndex_r4/Relational  mode=a=b  en=off  latency=0  dbl_ovrd=off  block_type=relational
  Relational2 : xbsIndex_r4/Relational  mode=a<b  en=off  latency=0  dbl_ovrd=off  block_type=relational
  Relational4 : xbsIndex_r4/Relational  mode=a=b  en=off  latency=0  dbl_ovrd=off  block_type=relational
  Scrambling LFSR : Subsystem
  edge : Subsystem
  " x_I" : Outport
  " x_Q" : Outport  Port=2
  wires:
  Constant2/1 -> Relational2/2
  Relational2/1 -> Scrambling LFSR/2(rst)
  Scrambling LFSR/1(Q) -> Logical/2
  Logical/1 -> Delay2/1
  Negate1/1 -> Mux1/3
  x_I/1 -> Negate1/1, Delay/1
  Sym Ind/1 -> edge/1(D), Relational2/1, Relational4/2, Relational1/2
  Negate/1 -> Logical/1
  Mux1/1 -> " x_I"/1
  Negate2/1 -> Mux2/3
  x_Q/1 -> Negate2/1, Delay1/1
  Mux2/1 -> " x_Q"/1
  Delay/1 -> Mux1/2
  Delay1/1 -> Mux2/2
  Delay2/1 -> Mux1/1, Mux2/1
  Constant1/1 -> Relational1/1
  Constant3/1 -> Relational4/1
  Relational1/1 -> Logical1/1
  Relational4/1 -> Logical1/2
  Logical1/1 -> Logical4/2
  [RX_PHY_MODE_11N_AC] -> Logical4/1
  Logical2/1 -> Scrambling LFSR/1(en)
  edge/1(Q) -> Logical2/2
  Logical4/1 -> Delay3/1
  Delay3/1 -> Logical2/1

== system wlan_phy_rx_pmd/Chan Est & EQ/Equalizer, Pilot Processing & PHY Mode Det/Phase Err Calc/Phase Calc ==
  note: ARCTAN outputs scaled radians:
      | Output [-1, +1] maps to actual
      | phase of [-pi, +pi]
  I : Inport
  Q : Inport  Port=2
  IQ Valid : Inport  Port=3
  Reset : Inport  Port=4
  "CORDIC 5.0 " : xbsIndex_r4/CORDIC 5.0   functional_selection=Arc_Tan  architectural_configuration=Parallel  pipelining_mode=Optimal  data_format=SignedFraction  phase_format=Scaled_Radians  input_width=16  output_width=16  round_mode=Truncate  iterations=0  precision=0  compensation_scaling=No_Scale_Compensation  coarse_rotation=on  aclken=off  aresetn=off  out_tready=off  cartesian_has_tuser=off  cartesian_has_tlast=off  cartesian_tuser_width=1  phase_has_tuser=off  phase_has_tlast=off  phase_tuser_width=1  out_tlast_behv=Null  flow_control=Blocking  optimize_goal=Resources  trim_axipin_name=on  ip_name=CORDIC  ip_version=5.0  dsptool_ready=true  ipcore_usecache=true  ipcore_useipmodelcache=true  wrapper_available=true  port_translation_map={ 'aclken' => 'en', 'aresetn' => 'rst' }  block_type=cordic_v5_0
      settings: infoedit=CORDIC 5.0
  Convert1 : xbsIndex_r4/Convert  gui_display_data_type=Fixed-point  arith_type="Signed  (2's comp)"  n_bits=17  bin_pt=15  float_type=Single  exp_bits=8  fraction_bits=24  quantization="Round  (unbiased: +/- Inf)"  overflow=Saturate  en=off  latency=1  dbl_ovrd=off  pipeline=off  block_type=convert
  Convert3 : xbsIndex_r4/Convert  gui_display_data_type=Fixed-point  arith_type="Signed  (2's comp)"  n_bits=17  bin_pt=15  float_type=Single  exp_bits=8  fraction_bits=24  quantization="Round  (unbiased: +/- Inf)"  overflow=Saturate  en=off  latency=1  dbl_ovrd=off  pipeline=off  block_type=convert
  Delay4 : xbsIndex_r4/Delay  rst=off  infoeditControl=Selection of Reset will increase slice count due to use of real FFs and instead of SRLs  en=off  latency=1  dbl_ovrd=off  reg_retiming=off  block_type=delay
  Gateway Out{1..5,8} (6 alike) : xbsIndex_r4/Gateway Out  inherit_from_input=off  hdl_port=off  timing_constraint=None  locs_specified=off  LOCs={}  UseAsDAC=off  DACChannel='1'  block_type=gatewayout
  Pilot Phase Calc : Scope  NumInputPorts=10
      settings: TimeRange=14000  YMin=0~-0.1~-0.2~-0.4~0~0~0~-0.2~-5~-5  YMax=80~0.1~0.2~0.4~80~1~1~0.2~5~5  SaveName=ScopeData21
  Register : xbsIndex_r4/Register  init=0  rst=on  en=on  dbl_ovrd=off  block_type=register
  phase err : Outport
  wires:
  Convert3/1 -> "CORDIC 5.0 "/2, Gateway Out1/1
  Convert1/1 -> "CORDIC 5.0 "/3, Gateway Out2/1
  "CORDIC 5.0 "/3 -> Register/1, Gateway Out4/1
  "CORDIC 5.0 "/2 -> Gateway Out3/1, Register/3
  Register/1 -> phase err/1
  Delay4/1 -> "CORDIC 5.0 "/1, Gateway Out8/1
  Q/1 -> Convert3/1
  I/1 -> Convert1/1
  IQ Valid/1 -> Delay4/1
  Reset/1 -> Gateway Out5/1, Register/2
  Gateway Out8/1 -> Pilot Phase Calc/1
  Gateway Out1/1 -> Pilot Phase Calc/2
  Gateway Out2/1 -> Pilot Phase Calc/3
  Gateway Out3/1 -> Pilot Phase Calc/4
  Gateway Out4/1 -> Pilot Phase Calc/5
  Gateway Out5/1 -> Pilot Phase Calc/6

== system wlan_phy_rx_pmd/Chan Est & EQ/Equalizer, Pilot Processing & PHY Mode Det/Phase Err Calc/Pilot Selection ==
  note: All PHY modes:
      | -Pilot tones in subcarriers [-21,-7,7,21]
      | -Subcarriers [-7,-21] are x_ind [57,43]
      | -One pilot per OFDM sybmol is opposite sign of other 3
      |
      | 11a/g:
      | -Opposite sign always in subcarrier 21
      |
      | 11n/ac:
      | -Syms 0:4: Same as a/g
      | -Syms 5:6: No pilot tones in HT-STF, HT-LTF
      | -Syms 7:N: Opposite sign pilot rotates across 4 subcarriers sequentially:
      |    7: 21
      |    8: 7
      |    9: -21
      |    10: -7
      |     ...
      | Logic uses 2LSB of sym index to select current negative pilot subcarrier
  x_valid : Inport
  x_ind : Inport  Port=2
  sym_ind : Inport  Port=3
  2LSB : xbsIndex_r4/Slice  nbits=2  boolean_output=off  mode=Lower Bit Location + Width  bit1=0  base1=MSB of Input  bit0=0  base0=LSB of Input  dbl_ovrd=off  block_type=slice
  Constant1 : xbsIndex_r4/Constant  const=21  gui_display_data_type=Fixed-point  arith_type=Unsigned  n_bits=6  bin_pt=0  preci_type=Single  exp_width=8  frac_width=24  explicit_period=off  period=8  equ=P=C  opselect=C  inp2=PCIN>>17  opr=+  inp1=P  carry=CIN  dbl_ovrd=off  block_type=constant
  Constant2 : xbsIndex_r4/Constant  const=7  gui_display_data_type=Fixed-point  arith_type=Unsigned  n_bits=6  bin_pt=0  preci_type=Single  exp_width=8  frac_width=24  explicit_period=off  period=8  equ=P=C  opselect=C  inp2=PCIN>>17  opr=+  inp1=P  carry=CIN  dbl_ovrd=off  block_type=constant
  Constant3 : xbsIndex_r4/Constant  const=64-7  gui_display_data_type=Fixed-point  arith_type=Unsigned  n_bits=6  bin_pt=0  preci_type=Single  exp_width=8  frac_width=24  explicit_period=off  period=8  equ=P=C  opselect=C  inp2=PCIN>>17  opr=+  inp1=P  carry=CIN  dbl_ovrd=off  block_type=constant
  Constant4 : xbsIndex_r4/Constant  const=64-21  gui_display_data_type=Fixed-point  arith_type=Unsigned  n_bits=6  bin_pt=0  preci_type=Single  exp_width=8  frac_width=24  explicit_period=off  period=8  equ=P=C  opselect=C  inp2=PCIN>>17  opr=+  inp1=P  carry=CIN  dbl_ovrd=off  block_type=constant
  Constant5 : xbsIndex_r4/Constant  const=6  gui_display_data_type=Fixed-point  arith_type=Unsigned  n_bits=3  bin_pt=0  preci_type=Single  exp_width=8  frac_width=24  explicit_period=off  period=8  equ=P=C  opselect=C  inp2=PCIN>>17  opr=+  inp1=P  carry=CIN  dbl_ovrd=off  block_type=constant
  Constant7 : xbsIndex_r4/Constant  const=5  gui_display_data_type=Fixed-point  arith_type=Unsigned  n_bits=3  bin_pt=0  preci_type=Single  exp_width=8  frac_width=24  explicit_period=off  period=1  equ=P=C  opselect=C  inp2=PCIN>>17  opr=+  inp1=P  carry=CIN  dbl_ovrd=off  block_type=constant
  Constant9 : xbsIndex_r4/Constant  const=6  gui_display_data_type=Fixed-point  arith_type=Unsigned  n_bits=3  bin_pt=0  preci_type=Single  exp_width=8  frac_width=24  explicit_period=off  period=1  equ=P=C  opselect=C  inp2=PCIN>>17  opr=+  inp1=P  carry=CIN  dbl_ovrd=off  block_type=constant
  Logical : xbsIndex_r4/Logical  logical_function=AND  inputs=3  en=off  latency=0  precision=Full  arith_type=Unsigned  n_bits=8  bin_pt=2  align_bp=on  dbl_ovrd=off  block_type=logical
      settings: xl_area=[0, 0, 0, 0, 0, 0, 0]  block_version=VER_STRING_GOES_HERE
  Logical1 : xbsIndex_r4/Logical  logical_function=OR  inputs=4  en=off  latency=0  precision=Full  arith_type=Unsigned  n_bits=8  bin_pt=2  align_bp=on  dbl_ovrd=off  block_type=logical
      settings: xl_area=[2 0 0 2 0 0 0]  block_version=VER_STRING_GOES_HERE
  Logical2 : xbsIndex_r4/Logical  logical_function=AND  inputs=2  en=off  latency=0  precision=Full  arith_type=Unsigned  n_bits=8  bin_pt=2  align_bp=on  dbl_ovrd=off  block_type=logical
      settings: xl_area=[0, 0, 0, 0, 0, 0, 0]  block_version=VER_STRING_GOES_HERE
  Logical3 : xbsIndex_r4/Logical  logical_function=OR  inputs=2  en=off  latency=0  precision=Full  arith_type=Unsigned  n_bits=16  bin_pt=0  align_bp=on  dbl_ovrd=off  block_type=logical
  Logical4 : xbsIndex_r4/Logical  logical_function=NAND  inputs=2  en=off  latency=0  precision=Full  arith_type=Unsigned  n_bits=16  bin_pt=0  align_bp=on  dbl_ovrd=off  block_type=logical
  Mux : xbsIndex_r4/Mux  inputs=4  en=off  latency=0  precision=Full  arith_type=Unsigned  n_bits=16  bin_pt=14  quantization=Truncate  overflow=Wrap  dbl_ovrd=off  block_type=mux
  Mux1 : xbsIndex_r4/Mux  inputs=2  en=off  latency=0  precision=Full  arith_type=Unsigned  n_bits=16  bin_pt=14  quantization=Truncate  overflow=Wrap  dbl_ovrd=off  block_type=mux
  Relational1 : xbsIndex_r4/Relational  mode=a>b  en=off  latency=0  dbl_ovrd=off  block_type=relational
      settings: xl_area=[2 0 0 3 0 0 0]  block_version=VER_STRING_GOES_HERE
  Relational{3..8} (6 alike) : xbsIndex_r4/Relational  mode=a=b  en=off  latency=0  dbl_ovrd=off  block_type=relational
      Relational4 settings: xl_area=[2 0 0 3 0 0 0]  block_version=VER_STRING_GOES_HERE
      Relational5 settings: xl_area=[2 0 0 3 0 0 0]  block_version=VER_STRING_GOES_HERE
      Relational6 settings: xl_area=[2 0 0 3 0 0 0]  block_version=VER_STRING_GOES_HERE
      Relational7 settings: xl_area=[2 0 0 3 0 0 0]  block_version=VER_STRING_GOES_HERE
  Pilot : Outport
  Negate : Outport  Port=2
  wires:
  Constant1/1 -> Relational5/1
  Constant2/1 -> Relational4/1
  Relational4/1 -> Logical1/1, Mux/2
  Relational5/1 -> Logical1/2, Mux1/2, Mux/5
  Relational6/1 -> Logical1/3, Mux/4
  Relational7/1 -> Logical1/4, Mux/3
  x_ind/1 -> Relational4/2, Relational5/2, Relational6/2, Relational7/2
  Logical1/1 -> Logical/2
  Constant4/1 -> Relational6/1
  Constant3/1 -> Relational7/1
  Logical/1 -> Pilot/1
  x_valid/1 -> Logical/1
  2LSB/1 -> Mux/1
  sym_ind/1 -> 2LSB/1, Relational1/1, Relational8/2, Relational3/2
  Constant5/1 -> Relational1/2
  Mux1/1 -> Negate/1
  [RX_PHY_MODE_11N_AC] -> Logical2/1, Logical4/2
  Relational1/1 -> Logical2/2
  Logical2/1 -> Mux1/1
  Constant7/1 -> Relational3/1
  Constant9/1 -> Relational8/1
  Relational3/1 -> Logical3/1
  Relational8/1 -> Logical3/2
  Logical3/1 -> Logical4/1
  Mux/1 -> Mux1/3
  Logical4/1 -> Logical/3

== system wlan_phy_rx_pmd/DSSS Rx/Demod/Header CRC/CRC LFSR ==
  Bit : Inport
  Bit Valid : Inport  Port=2
  Concat : xbsIndex_r4/Concat  num_inputs=16  dbl_ovrd=off  block_type=concat
  Inverter : xbsIndex_r4/Inverter  en=off  latency=0  dbl_ovrd=off  block_type=inv
  Logical : xbsIndex_r4/Logical  logical_function=XOR  inputs=2  en=off  latency=0  precision=Full  arith_type=Unsigned  n_bits=16  bin_pt=0  align_bp=on  dbl_ovrd=off  block_type=logical
  Logical1 : xbsIndex_r4/Logical  logical_function=XOR  inputs=2  en=off  latency=0  precision=Full  arith_type=Unsigned  n_bits=16  bin_pt=0  align_bp=on  dbl_ovrd=off  block_type=logical
  Logical2 : xbsIndex_r4/Logical  logical_function=XOR  inputs=2  en=off  latency=0  precision=Full  arith_type=Unsigned  n_bits=16  bin_pt=0  align_bp=on  dbl_ovrd=off  block_type=logical
  Register{1..16} (16 alike) : xbsIndex_r4/Register  init=1  rst=on  en=on  dbl_ovrd=off  block_type=register
      settings: xl_area=[0, 0, 0, 0, 0, 0, 0]  block_version=VER_STRING_GOES_HERE
  CRC16 : Outport
  wires:
  Register1/1 -> Register2/1, Concat/1
  Register2/1 -> Register3/1, Concat/2
  Register3/1 -> Register4/1, Concat/3
  Register5/1 -> Logical1/2, Concat/5
  Register6/1 -> Register7/1, Concat/6
  Register7/1 -> Register8/1, Concat/7
  Register4/1 -> Register5/1, Concat/4
  Bit Valid/1 -> Register1/3, Register2/3, Register3/3, Register4/3, Register5/3, Register6/3, Register7/3, Register8/3, Register9/3, Register10/3, Register11/3, Register14/3, Register15/3, Register16/3, Register12/3, Register13/3
  [DSSS_RX_RESET] -> Register1/2, Register7/2, Register8/2, Register10/2, Register11/2, Register14/2, Register15/2, Register12/2, Register13/2, Register16/2, Register9/2, Register6/2, Register5/2, Register4/2, Register3/2, Register2/2
  Register8/1 -> Register9/1, Concat/8
  Register9/1 -> Register10/1, Concat/9
  Register10/1 -> Register11/1, Concat/10
  Bit/1 -> Logical/2
  Register11/1 -> Register14/1, Concat/11
  Register14/1 -> Logical2/2, Concat/12
  Register15/1 -> Register16/1, Concat/13
  Register16/1 -> Register12/1, Concat/14
  Register12/1 -> Register13/1, Concat/15
  Logical2/1 -> Register15/1
  Logical1/1 -> Register6/1
  Logical/1 -> Register1/1, Logical1/1, Logical2/1
  Register13/1 -> Concat/16, Logical/1
  Concat/1 -> Inverter/1
  Inverter/1 -> CRC16/1

== system wlan_phy_rx_pmd/DSSS Rx/Demod/Sync/S-R Latch ==
  S : Inport
  R : Inport  Port=2
  Constant1 : xbsIndex_r4/Constant  const=1  gui_display_data_type=Fixed-point  arith_type=Boolean  n_bits=1  bin_pt=0  preci_type=Single  exp_width=8  frac_width=24  explicit_period=off  period=1  equ=P=C  opselect=C  inp2=PCIN>>17  opr=+  inp1=P  carry=CIN  dbl_ovrd=off  block_type=constant
      settings: block_version=VER_STRING_GOES_HERE
  Convert1 : xbsIndex_r4/Convert  gui_display_data_type=Boolean  arith_type=Boolean  n_bits=1  bin_pt=0  float_type=Single  exp_bits=8  fraction_bits=24  quantization=Truncate  overflow=Wrap  en=off  latency=0  dbl_ovrd=off  pipeline=off  block_type=convert
  Convert2 : xbsIndex_r4/Convert  gui_display_data_type=Boolean  arith_type=Boolean  n_bits=1  bin_pt=0  float_type=Single  exp_bits=8  fraction_bits=24  quantization=Truncate  overflow=Wrap  en=off  latency=0  dbl_ovrd=off  pipeline=off  block_type=convert
  Register2 : xbsIndex_r4/Register  init=0  rst=on  en=on  dbl_ovrd=off  block_type=register
      settings: xl_area=[1 1 0 0 0 0 0]  block_version=VER_STRING_GOES_HERE
  Q : Outport
  wires:
  Convert2/1 -> Register2/3
  Convert1/1 -> Register2/2
  Constant1/1 -> Register2/1
  R/1 -> Convert1/1
  Register2/1 -> Q/1
  S/1 -> Convert2/1
